simulink subsystem "ButtonStatesRF"  (R2023b)
settings: Solver=VariableStepAuto  StartTime=0.0  StopTime=10.0  RelTol=1e-3
undefined symbols (not defined in the file or in its folder): eStateName
libraries: simulink x5
(part 2 of 2)

== stateflow chart ButtonStatesRF/ButtonStates/buttonFlowChart ==
  state DiagChecker [parallel]
    settings: executionOrder=4
    state CheckHazard [parallel] [commented out]
      state HazardON [commented out]
      state HazardOFF [commented out]
      (default) -> HazardOFF [commented out]
      HazardOFF -> HazardON [commented out] : [hasChangedTo(hazard,true)]
      (junction 4891) -> HazardOFF [commented out]
      (junction 4893) -> (junction 4891) [commented out] : {send(HAZARD, Flow);}
      HazardON -> (junction 4893) [commented out] : [hasChangedTo(ignition,true)]
      (junction 4896) -> (junction 4891) [commented out]
      (junction 4893) -> (junction 4895) [commented out] : [hazard==false]
      (junction 4895) -> (junction 4896) [commented out]
      HazardON -> (junction 4895) [commented out] : [hasChangedTo(hazard,false)]
    state CheckStateReset [parallel]
      settings: executionOrder=1
      state ResetChk
      (default) -> ResetChk
      ResetChk -> (junction 5069) : [hasChangedTo(stateResetFlg,true)]
      (junction 5069) -> (junction 5067)
      (junction 5067) -> ResetChk : {send(RESET, Flow);}
    state CheckVBAT [parallel] [commented out]
      state setHigh [commented out]
        en:
        DASH_RCM_Set_Switch = eButton.pressed;
        en,du:
        setDur = elapsed(sec);
      state setLow [commented out]
        en:
        DASH_RCM_Set_Switch = eButton.released;
      (default) -> DiagChecker.CheckVBAT.setLow [commented out]
      DiagChecker.CheckVBAT.setLow -> DiagChecker.CheckVBAT.setHigh [commented out] : [hasChangedTo(set,true)]
      (junction 4939) -> DiagChecker.CheckVBAT.setLow [commented out]
      (junction 4940) -> (junction 4939) [commented out] : {send(SET,Flow);}
      (junction 4941) -> (junction 4940) [commented out] : [setDur*1000>=BLU_SetDtctMin_Tms && ...
          | setDur*1000 <=BLU_SetDtctMax_Tms ]
      DiagChecker.CheckVBAT.setHigh -> (junction 4941) [commented out] : [hasChangedTo(set,false)]
      (junction 4945) -> (junction 4939) [commented out]
      (junction 4941) -> (junction 4944) [commented out]
      DiagChecker.CheckVBAT.setHigh -> (junction 4942) [commented out] : after(3,sec)
      (junction 4942) -> (junction 4943) [commented out]
      (junction 4944) -> (junction 4943) [commented out] : [setDur*1000>=BLU_LngSetDtctMin_Tms && ...
          | setDur*1000 <=BLU_LngSetDtctMax_Tms ]
      (junction 4943) -> (junction 4945) [commented out] : {send(longSET,Flow);}
      (junction 4944) -> (junction 4947) [commented out]
      (junction 4946) -> (junction 4945) [commented out]
      (junction 4947) -> (junction 4946) [commented out]
    state CheckExit [parallel] [commented out]
      state exitHigh [commented out]
        en:
        DASH_RCM_Exit_Switch = eButton.pressed;
        en,du:
        exitDur = elapsed(sec);
      state exitLow [commented out]
        en:
        DASH_RCM_Exit_Switch = eButton.released;
      (default) -> DiagChecker.CheckExit.exitLow [commented out]
      DiagChecker.CheckExit.exitLow -> DiagChecker.CheckExit.exitHigh [commented out] : [hasChangedTo(exitButton,true)]
      (junction 4966) -> DiagChecker.CheckExit.exitLow [commented out]
      (junction 4967) -> (junction 4966) [commented out] : {send(EXIT,Flow);}
      (junction 4968) -> (junction 4967) [commented out] : [exitDur*1000>=BLU_ExitDtctMin_Tms && ...
          | exitDur*1000 <=BLU_ExitDtctMax_Tms ]
      DiagChecker.CheckExit.exitHigh -> (junction 4968) [commented out] : [hasChangedTo(exitButton,false)]
      (junction 4969) -> (junction 4966) [commented out]
      (junction 4968) -> (junction 4971) [commented out]
      (junction 4970) -> (junction 4969) [commented out] : {send(longEXIT,Flow);}
      (junction 4971) -> (junction 4970) [commented out] : [exitDur*1000>=BLU_LngExitDtctMin_Tms && ...
          | exitDur*1000 <=BLU_LngExitDtctMax_Tms ]
      (junction 4972) -> (junction 4969) [commented out]
      (junction 4971) -> (junction 4973) [commented out]
      (junction 4973) -> (junction 4972) [commented out]
    state CheckCC [parallel] [commented out]
      state setHigh [commented out]
        en:
        DASH_CC_Master_Switch = eButton.pressed;
        en,du:
        ccDur = elapsed(sec);
      state setLow [commented out]
        en:
        DASH_CC_Master_Switch = eButton.released;
      (default) -> DiagChecker.CheckCC.setLow [commented out]
      (junction 4990) -> DiagChecker.CheckCC.setHigh [commented out] : [ECU_CC_Feature==1]
      DiagChecker.CheckCC.setLow -> (junction 4990) [commented out] : [hasChangedTo(cc,true)]
      (junction 4991) -> DiagChecker.CheckCC.setLow [commented out] : {send(CC,Flow);}
      (junction 4992) -> (junction 4991) [commented out] : [ccDur >=BLU_CCDtctMin_Tms && ...
          | ccDur <=BLU_CCDtctMax_Tms ]
      DiagChecker.CheckCC.setHigh -> (junction 4992) [commented out] : [hasChangedTo(cc,false)]
    state CheckResume [parallel] [commented out]
      state setHigh [commented out]
        en:
        DASH_Cc_Resume_Switch = eButton.pressed;
        en,du:
        resumeDur = elapsed(sec)
      state setLow [commented out]
        en:
        DASH_Cc_Resume_Switch = eButton.released;
      (default) -> DiagChecker.CheckResume.setLow [commented out]
      DiagChecker.CheckResume.setLow -> (junction 5002) [commented out] : [hasChangedTo(resume,true)]
      (junction 5002) -> DiagChecker.CheckResume.setHigh [commented out] : [ECU_CC_Feature==1]
      (junction 5003) -> DiagChecker.CheckResume.setLow [commented out] : {send(RESUME,Flow);}
      (junction 5004) -> (junction 5003) [commented out] : [resumeDur >=BLU_ResDtctMin_Tms && ...
          | resumeDur <=BLU_ResDtctMax_Tms ]
      DiagChecker.CheckResume.setHigh -> (junction 5004) [commented out] : [hasChangedTo(resume,false)]
    state CheckCCSet [parallel] [commented out]
      state setHigh [commented out]
        en:
        DASH_Cc_Set_Switch = eButton.pressed;
      state setLow [commented out]
        en:
        DASH_Cc_Set_Switch = eButton.released;
      (default) -> DiagChecker.CheckCCSet.setLow [commented out]
      DiagChecker.CheckCCSet.setLow -> (junction 5014) [commented out] : [hasChangedTo(ccSet,true)]
      (junction 5014) -> DiagChecker.CheckCCSet.setHigh [commented out] : [ECU_CC_Feature==1]
      (junction 5015) -> DiagChecker.CheckCCSet.setLow [commented out] : {send(longCC,Flow);}
      DiagChecker.CheckCCSet.setHigh -> (junction 5015) [commented out] : [duration(set==true,msec) >=BLU_LngCCDtctMin_Tms && ...
          | duration(set==true,msec) <=BLU_LngCCDtctMax_Tms ]
      (junction 5016) -> DiagChecker.CheckCCSet.setLow [commented out] : [hasChangedTo(cc,false)]
          | {send(CCSet,Flow);}
      DiagChecker.CheckCCSet.setHigh -> (junction 5016) [commented out] : [duration(set==true,msec) >=BLU_CCSetDtctMin_Tms && ...
          | duration(set==true,msec) <=BLU_CCSetDtctMax_Tms ]
  state Flow [parallel]
    settings: executionOrder=3
    state ButtonTriggeredMenu
      (history)
      state MainScreen
        state RemoteControMode
          en:
          currentState = eStateName.REMOTE_CONTROL_MODE;
          DASH_RCM_Ready_Switch = eButton.pressed;
        state WaitForButton
          en:
          currentState = eStateName.MAIN_SCREEN;
          QuickSelectorDbg = 0;
        WaitForButton -> RemoteControMode [order 4] : [CCU_RCM_Feature == 1 && ...
            | CCU_RCM_Ready_Status == 1]
        RemoteControMode -> WaitForButton : longEXIT
        (default) -> WaitForButton
        2590 -> WaitForButton
        WaitForButton -> (junction 105) [order 1] : SET
        (junction 105) -> 2593 [order 2]
        (junction 105) -> (junction 107) [order 1] : [ECU_SL_LED == 1 && ...
            | ECU_CC_Feature == 0]
            | {DASH_CC_Master_Switch = eButton.pressed;}
        (junction 107) -> WaitForButton
        WaitForButton -> (junction 60) [order 2] : UP
        (junction 70) -> WaitForButton
        WaitForButton -> (junction 84) [order 3] : DOWN
        (junction 60) -> (junction 70) [order 1] : [ECU_SL_Status == 1  && ...
            | ECU_CC_Feature == 0]
            | {DASH_Cc_Resume_Switch  = eButton.pressed;}
        (junction 84) -> (junction 70) [order 1] : [ECU_SL_Status == 1 && ...
            |  ECU_CC_Feature == 0]
            | {DASH_Cc_Set_Switch  = eButton.pressed;}
        (junction 60) -> 2602 [order 2] : [rideMode != eRideMode.track]
        (junction 90) -> (junction 70)
        (junction 84) -> (junction 88) [order 2]
        (junction 88) -> (junction 90) [order 1] : [rideMode == eRideMode.track]
            | {startLapOrLapTimer();}
        (junction 88) -> 2605 [order 2] : [rideMode != eRideMode.track]
      state Menu
        (history)
        state MainSubMenu
          (history)
          state LapTimer
            en:
            currentState = eStateName.LAP_TIMER;
            state LapTimerEntry
            state GreyedOut
            (default) -> (junction 222)
            (junction 222) -> LapTimerEntry [order 1] : [rideMode == eRideMode.track]
            (junction 222) -> (junction 225) [order 2]
            (junction 225) -> GreyedOut
          state Lap_TimerV2
            (history)
            state LapTimerLoop
              en:
              currentState = eStateName.RESET_LAPS;
            state RoundTimerMenu
              en:
              currentState = eStateName.RESET_LAPS_RND_TMR;
            state ResetLaps
              state ResetLapsMenu
                state Keep
                  en:
                  currentState = eStateName.RESETLAPS_KEEP;
                state Reset
                  en:
                  currentState = eStateName.RESETLAPS_RESET;
                  //updateRoundTimeData();
                (default) -> Flow.ButtonTriggeredMenu.Menu.MainSubMenu.Lap_TimerV2.ResetLaps.ResetLapsMenu.Keep
                Flow.ButtonTriggeredMenu.Menu.MainSubMenu.Lap_TimerV2.ResetLaps.ResetLapsMenu.Keep -> Flow.ButtonTriggeredMenu.Menu.MainSubMenu.Lap_TimerV2.ResetLaps.ResetLapsMenu.Reset [order 1] : DOWN || UP
                Flow.ButtonTriggeredMenu.Menu.MainSubMenu.Lap_TimerV2.ResetLaps.ResetLapsMenu.Reset -> Flow.ButtonTriggeredMenu.Menu.MainSubMenu.Lap_TimerV2.ResetLaps.ResetLapsMenu.Keep [order 1] : DOWN || UP
              Flow.ButtonTriggeredMenu.Menu.MainSubMenu.Lap_TimerV2.ResetLaps.ResetLapsMenu.Keep -> (junction 8239) [order 2] : SET
              (junction 8239) -> 8241
              ResetLapsMenu -> 8241 : EXIT
              (junction 8240) -> 8241
              Flow.ButtonTriggeredMenu.Menu.MainSubMenu.Lap_TimerV2.ResetLaps.ResetLapsMenu.Reset -> (junction 8240) [order 2] : SET
                  | {BLU_FnRLResetRndTmr();}
            (junction 8201) -> 8211
            Flow.ButtonTriggeredMenu.Menu.MainSubMenu.Lap_TimerV2.LapTimerLoop -> 8211 [order 2] : EXIT
            RoundTimerMenu -> (junction 8201) [order 3] : EXIT
            (default) -> (junction 8202)
            (junction 8202) -> Flow.ButtonTriggeredMenu.Menu.MainSubMenu.Lap_TimerV2.LapTimerLoop : {roundTimeCounter = 0;
                | copyRoundTimeData();
                | updateRoundTimeData();}
            8212 -> (junction 8202)
            (junction 8203) -> Flow.ButtonTriggeredMenu.Menu.MainSubMenu.Lap_TimerV2.LapTimerLoop
            (junction 8204) -> (junction 8203) [order 2]
            RoundTimerMenu -> (junction 8204) [order 1] : UP
            (junction 8204) -> (junction 8205) [order 1] : [BLU_RND_TMR_LOOP ==1]
            Flow.ButtonTriggeredMenu.Menu.MainSubMenu.Lap_TimerV2.LapTimerLoop -> (junction 8206) [order 4] : UP
            (junction 8206) -> RoundTimerMenu : [BLU_RND_TMR_LOOP ==1]
                | {BLU_FnLapTmrScrllUP();}
            (junction 8208) -> (junction 8204) [order 2]
            (junction 8205) -> (junction 8207) : {BLU_FnRLRndTmrUp();}
            (junction 8207) -> RoundTimerMenu
            Flow.ButtonTriggeredMenu.Menu.MainSubMenu.Lap_TimerV2.LapTimerLoop -> Flow.ButtonTriggeredMenu.Menu.MainSubMenu.Lap_TimerV2.ResetLaps [order 1] : SET
            8213 -> Flow.ButtonTriggeredMenu.Menu.MainSubMenu.Lap_TimerV2.LapTimerLoop
            Flow.ButtonTriggeredMenu.Menu.MainSubMenu.Lap_TimerV2.LapTimerLoop -> (junction 8210) [order 3] : DOWN
            (junction 8209) -> (junction 8207) : {BLU_FnRLRndTmrDwn();}
            RoundTimerMenu -> (junction 8208) [order 2] : DOWN
            (junction 8208) -> (junction 8209) [order 1] : [BLU_RND_TMR_LOOP ==1]
            (junction 8210) -> RoundTimerMenu : [BLU_RND_TMR_LOOP ==1]
                | {BLU_FnLapTmrScrllDOWN();}
          state MotorCycleSubMenu
            (history)
            state MotorCycleSubMenu1
              (history)
              state RideMode
                en:
                currentState = eStateName.MOTORCYCLE_RIDEMODE;
              state ABS
                en:
                currentState = eStateName.MOTORCYCLE_ABS;
              state MTC
                en:
                currentState = eStateName.MOTORCYCLE_MTC;
              state LaunchControl
                en:
                currentState = eStateName.MOTORCYCLE_LAUNCHCONTROL;
              (default) -> (junction 5718)
              (junction 5718) -> (junction 5719)
              (junction 5719) -> RideMode [order 1] : [variant !=eBikeVariant.duke200]
              RideMode -> 5733 [order 2] : SET
              (junction 5731) -> (junction 5718)
              (junction 5719) -> (junction 5723) [order 2]
              RideMode -> (junction 5720) [order 3] : UP
              (junction 5721) -> RideMode
              5734 -> (junction 5721)
              (junction 5720) -> (junction 5727)
              (junction 5722) -> (junction 5721) : [variant !=eBikeVariant.duke200]
              RideMode -> ABS [order 1] : DOWN
              ABS -> (junction 5722) [order 1] : UP
              (junction 5723) -> ABS
              ABS -> 5735 [order 2] : SET
              (junction 5724) -> ABS
              5736 -> (junction 5724)
              (junction 5728) -> (junction 5724) [order 2]
              MTC -> (junction 5724) [order 2] : UP
              ABS -> (junction 5726) [order 3] : DOWN
              MTC -> (junction 5725) [order 1] : SET
              (junction 5725) -> 5737 : [ABS_TC_MTC_Mode_Feature == eTCMode.TCMode_TC || ...
                  | ABS_TC_MTC_Mode_Feature == eTCMode.TCMode_MTC || ...
                  | ABS_TC_MTC_Mode_Feature == eTCMode.TCMode_TC_Demo || ...
                  | ABS_TC_MTC_Mode_Feature == eTCMode.TCMode_MTC_Demo ]
              5738 -> (junction 5726)
              (junction 5726) -> MTC [order 1] : [ABS_TC_MTC_Mode_Feature !=eTCMode.TCMode_OFF && ...
                  | variant !=eBikeVariant.duke200]
              (junction 5726) -> (junction 5729) [order 2]
              MTC -> (junction 5729) [order 3] : DOWN
              (junction 5728) -> MTC [order 1] : [ABS_TC_MTC_Mode_Feature !=eTCMode.TCMode_OFF]
              (junction 5727) -> (junction 5728) [order 2]
              (junction 5727) -> (junction 5730) [order 1] : [rideMode == eRideMode.rain && ...
                  | ABS_Launch_Control_Feature == 1]
              LaunchControl -> (junction 5728) [order 1] : UP
              (junction 5729) -> LaunchControl [order 1] : [rideMode == eRideMode.rain && ...
                  | ABS_Launch_Control_Feature == 1 && ...
                  | variant !=eBikeVariant.duke200]
              (junction 5729) -> (junction 5732) [order 2]
              LaunchControl -> 5739 [order 2] : SET
              (junction 5730) -> LaunchControl
              (junction 5732) -> (junction 5731)
              LaunchControl -> (junction 5732) [order 3] : DOWN
            state RideModeSubMenu
              (history)
              state RideModeSubMenu1
                (history)
                state Track
                  en:
                  currentState = eStateName.MC_RIDEMODE_TRACK;
                state Street
                  en:
                  currentState = eStateName.MC_RIDEMODE_STREET;
                state Rain
                  en:
                  currentState = eStateName.MC_RIDEMODE_RAIN;
                Track -> (junction 5829) [order 1]
                (junction 5830) -> Track
                (junction 5829) -> (junction 5832) [order 2] : DOWN
                (junction 5833) -> (junction 5830)
                Track -> Street [order 2] : UP
                Street -> Track [order 3] : DOWN
                Street -> Rain [order 2] : UP
                Rain -> Street [order 1] : DOWN
                (junction 5832) -> Rain
                Rain -> (junction 5833) [order 3] : UP
              state TrackCloseThrottle
                en:
                currentState = eStateName.MC_CLOSETHROTTLE;
              state KillSwitch
                en:
                currentState = eStateName.MC_DEACTIVATE_KILLSWITCH;
              state StreetCloseThrottle
                en:
                currentState = eStateName.MC_CLOSETHROTTLE;
              state RainCloseThrottle
                en:
                currentState = eStateName.MC_CLOSETHROTTLE;
              (junction 5829) -> (junction 5781) [order 1] : SET
              (junction 5781) -> (junction 5782) [order 1] : [killSwitch == eSwitch.closed]
              (junction 5782) -> TrackCloseThrottle : {DASH_TC_Mode_Request = 0;
                  | DASH_Drive_Mode_Request = 0;}
              (junction 5781) -> KillSwitch [order 2]
              TrackCloseThrottle -> (junction 5784) [order 2] : after(5,sec)
              TrackCloseThrottle -> (junction 5785) [order 1] : after(500,msec)
              KillSwitch -> (junction 5783) : [after(1,sec)]
              (junction 5784) -> (junction 5783)
              (junction 5785) -> (junction 5784) : [ABS_TC_Mode_Response == 0 && ...
                  | ECU_Drive_Mode_Response == 0]
                  | {rideMode = eRideMode.track;}
              (junction 5783) -> (junction 5790)
              (junction 5787) -> KillSwitch [order 2]
              (junction 5786) -> Street
              5796 -> (junction 5786)
              Street -> (junction 5787) [order 1] : SET
              (junction 5787) -> (junction 5788) [order 1] : [killSwitch == eSwitch.closed]
              (junction 5788) -> StreetCloseThrottle : {DASH_TC_Mode_Request = 0;
                  | DASH_Drive_Mode_Request = 0;}
              StreetCloseThrottle -> (junction 5789) [order 1] : after(500,msec)
              (junction 5794) -> (junction 5787) [order 2]
              (junction 5789) -> (junction 5792) : [ABS_TC_Mode_Response == 0 && ...
                  | ECU_Drive_Mode_Response == 0]
                  | {rideMode = eRideMode.street;}
              StreetCloseThrottle -> (junction 5791) [order 2] : after(5,sec)
              (junction 5791) -> (junction 5790)
              (junction 5792) -> (junction 5791)
              (junction 5790) -> 5797
              RainCloseThrottle -> (junction 5791) [order 2] : after(5,sec)
              (junction 5793) -> (junction 5792) : [ABS_TC_Mode_Response == 2 && ...
                  | ECU_Drive_Mode_Response == 2]
                  | {rideMode = eRideMode.rain;}
              RainCloseThrottle -> (junction 5793) [order 1] : after(500,msec)
              Rain -> (junction 5794) [order 2] : SET
              (junction 5794) -> (junction 5795) [order 1] : [killSwitch == eSwitch.closed]
              (junction 5795) -> RainCloseThrottle : {DASH_TC_Mode_Request = 2;
                  | DASH_Drive_Mode_Request = 2;}
              RideModeSubMenu1 -> 5797 : EXIT
            state ABSSubMenu
              (history)
              state ABSMenu1
                (history)
                state ABSRoad
                  en:
                  currentState = eStateName.ABS_ROAD;
                state ABSSuperMoto
                  en:
                  currentState = eStateName.ABS_SUPERMOTO;
                (default) -> ABSRoad
                ABSRoad -> (junction 5874) [order 1] : DOWN || UP
                ABSSuperMoto -> ABSRoad [order 1] : DOWN || UP
                (junction 5874) -> ABSSuperMoto : [ABS_Mode_Feature == 1]
              state SetABStoRoad
                en:
                DASH_ABS_Mode_Request = eABSMode.road;
              state SetABStoSupermoto
                en:
                DASH_ABS_Mode_Request = eABSMode.supermoto;
              SetABStoRoad -> (junction 5852) [order 1] : after(500,msec)
              ABSRoad -> SetABStoRoad [order 2] : SET
              (junction 5853) -> ABSMenu1
              (junction 5852) -> (junction 5855) : [ABS_ABS_Mode_Response == eABSMode.road]
              5857 -> (junction 5853)
              SetABStoRoad -> (junction 5854) [order 2] : after(5,sec)
              (junction 5855) -> (junction 5854)
              ABSMenu1 -> 5858 : EXIT
              (junction 5854) -> 5858
              SetABStoSupermoto -> (junction 5854) [order 2] : after(5,sec)
              (junction 5856) -> (junction 5855) : [ABS_ABS_Mode_Response == eABSMode.supermoto]
              SetABStoSupermoto -> (junction 5856) [order 1] : after(500,msec)
              ABSSuperMoto -> SetABStoSupermoto [order 2] : SET
            state MTCSubMenu
              (history)
              state MTCMenuOptions
                (history)
                state MTC_Off
                  en:
                  currentState = eStateName.MTCMODE_OFF;
                state MTC_On
                  en:
                  currentState = eStateName.MTCMODE_ON;
                (default) -> MTC_Off
                MTC_Off -> MTC_On [order 1] : DOWN || UP
                MTC_On -> MTC_Off [order 1] : DOWN || UP
              state MTC_Message
                en:
                currentState = eStateName.MTC_KEEP_OFF_PRESS;
              state MTC_Release
                en:
                currentState = eStateName.MTC_OFF_RELEASE;
              state MTC_Message1
                en:
                currentState = eStateName.MTC_KEEP_ON_PRESS;
              state MTC_Release1
                en:
                currentState = eStateName.MTC_ON_RELEASE;
              (junction 5884) -> (junction 5885)
              (junction 5885) -> (junction 5886) [order 1] : [ABS_TC_MTC_Mode_Feature == eTCMode.TCMode_TC || ...
                  | ABS_TC_MTC_Mode_Feature == eTCMode.TCMode_TC_Demo ]
              (junction 5885) -> (junction 5887) [order 2]
              5891 -> (junction 5884)
              (junction 5886) -> (junction 5888) : {currentState = eStateName.MTCMODE_TC;}
              (junction 5887) -> (junction 5888) : {currentState = eStateName.MTCMODE_MTC;}
              (junction 5888) -> MTCMenuOptions
              MTC_Off -> MTC_Message [order 2] : [set]
              MTC_Message -> MTC_Release [order 2] : after(3,sec)
              MTC_Message -> (junction 5889) [order 1] : [!set]
              MTC_Release -> (junction 5890) : [!set]
                  | {DASH_MTC_Off_Switch = eButton.pressed;}
              MTCMenuOptions -> 5892 : EXIT
              (junction 5889) -> 5892
              (junction 5890) -> (junction 5889)
              MTC_Message1 -> (junction 5889) [order 1] : [!set]
              MTC_Release1 -> (junction 5890) : [!set]
                  | {DASH_MTC_Off_Switch = eButton.released;}
              MTC_On -> MTC_Message1 [order 2] : [set]
              MTC_Message1 -> MTC_Release1 [order 2] : after(3,sec)
            state LaunchControlSubMenu
              state LaunchControlSubMenu1
                (history)
                state LaunchControlON
                  en:
                  currentState = eStateName.MC_LAUNCHCONTROL_ON;
                state LaunchControlOFF
                  en:
                  currentState = eStateName.MC_LAUNCHCONTROL_OFF;
                LaunchControlON -> LaunchControlOFF [order 2] : DOWN || UP
                LaunchControlOFF -> LaunchControlON [order 1] : DOWN || UP
              5926 -> LaunchControlON
              LaunchControlON -> (junction 5923) [order 1] : SET
              (junction 5923) -> (junction 5924) : {BLU_FnLaunchControlON();}
              (junction 5925) -> (junction 5924) : {BLU_FnLaunchControlOFF();}
              (junction 5924) -> 5927
              LaunchControlOFF -> (junction 5925) [order 2] : SET
              LaunchControlSubMenu1 -> 5927 : EXIT
            5681 -> 5683
            5680 -> 5684
            5679 -> 5682
            5685 -> MotorCycleSubMenu1
            5686 -> 5687
            5688 -> (junction 5675)
            (junction 5675) -> (junction 5676)
            5689 -> 5690
            5691 -> (junction 5676)
            (junction 5676) -> (junction 5677)
            MotorCycleSubMenu1 -> 5692 : EXIT
            5693 -> 5694
            5695 -> (junction 5677)
            (junction 5677) -> (junction 5678)
            (junction 5678) -> MotorCycleSubMenu1
            5696 -> (junction 5678)
            5697 -> 5698
          state Motorcycle
            en:
            currentState = eStateName.MOTORCYCLE;
          state BikeInfoSubMenu
            (history)
            state BikeInfoSubMenuMain
              (history)
              state WaterTemp
                en:
                currentState = eStateName.WATER_TEMPERATURE;
              state FuelRange
                en:
                currentState = eStateName.FUEL_RANGE;
              state Battery
                en:
                currentState = eStateName.BATTERY;
              state Odometer
                en:
                currentState = eStateName.ODOMETER;
              state Service
                en:
                currentState = eStateName.SERVICE;
              state Warnings
                en:
                currentState = eStateName.WARNINGS;
              (default) -> (junction 5540)
              WaterTemp -> (junction 5541) [order 2] : UP
              (junction 5540) -> WaterTemp
              (junction 5543) -> (junction 5540)
              (junction 5541) -> (junction 5542)
              WaterTemp -> FuelRange [order 1] : DOWN
              FuelRange -> WaterTemp [order 2] : UP
              FuelRange -> Battery [order 1] : DOWN
              Battery -> FuelRange [order 2] : UP
              Battery -> Odometer [order 1] : DOWN
              Odometer -> Battery [order 2] : UP
              Odometer -> Service [order 1] : DOWN
              Service -> Odometer [order 2] : UP
              Service -> Warnings [order 1] : DOWN
              Warnings -> Service [order 1] : UP
              (junction 5542) -> Warnings
              Warnings -> (junction 5543) [order 3] : DOWN
            state WarningsSubMenu
              en:
              currentState = eStateName.WARNINGS_MENU;
            5526 -> (junction 5540)
            5527 -> (junction 5540)
            BikeInfoSubMenuMain -> 5528 : EXIT
            WarningsSubMenu -> (junction 5523) [order 2] : UP
            (junction 5523) -> (junction 5524) : {BLU_FnBIWarningsUp();}
            WarningsSubMenu -> (junction 5542) [order 1] : EXIT
            (junction 5524) -> WarningsSubMenu
            (junction 5525) -> (junction 5524) : {BLU_FnBIWarningsDwn();}
            Warnings -> WarningsSubMenu [order 2] : SET
            WarningsSubMenu -> (junction 5525) [order 3] : DOWN
          state BikeInfo
            en:
            currentState = eStateName.BIKE_INFO;
          state TripInfoSubMenu
            (history)
            state TripInfoSubMenuMain
              (history)
              state TRIP1
                en:
                currentState = eStateName.TRIP_INFO_TRIP_1;
              state TRIP2
                en:
                currentState = eStateName.TRIP_INFO_TRIP_2;
              (default) -> (junction 5602)
              5605 -> (junction 5602)
              (junction 5602) -> TRIP1
              TRIP1 -> 5606 [order 2] : SET
              (junction 5603) -> TRIP1
              TRIP1 -> (junction 5604) [order 1] : DOWN || UP
              5607 -> (junction 5603)
              5608 -> (junction 5604)
              (junction 5604) -> TRIP2
              TRIP2 -> (junction 5603) [order 1] : DOWN || UP
              TRIP2 -> 5609 [order 2] : SET
            state Trip1
              state Trip1SubMenu
                (history)
                state SetAsOverview
                  en:
                  currentState = eStateName.TRIP_1_SET_AS_OVERVIEW;
                state ResetTrip
                  en:
                  currentState = eStateName.TRIP_1_RESET_TRIP;
                Flow.ButtonTriggeredMenu.Menu.MainSubMenu.TripInfoSubMenu.Trip1.Trip1SubMenu.SetAsOverview -> 5635 [order 2] : SET
                    | {BLU_FnTISetAsOvwTrip1();}
                Flow.ButtonTriggeredMenu.Menu.MainSubMenu.TripInfoSubMenu.Trip1.Trip1SubMenu.ResetTrip -> Flow.ButtonTriggeredMenu.Menu.MainSubMenu.TripInfoSubMenu.Trip1.Trip1SubMenu.SetAsOverview [order 1] : DOWN || UP
                Flow.ButtonTriggeredMenu.Menu.MainSubMenu.TripInfoSubMenu.Trip1.Trip1SubMenu.SetAsOverview -> Flow.ButtonTriggeredMenu.Menu.MainSubMenu.TripInfoSubMenu.Trip1.Trip1SubMenu.ResetTrip [order 1] : DOWN || UP
                Flow.ButtonTriggeredMenu.Menu.MainSubMenu.TripInfoSubMenu.Trip1.Trip1SubMenu.ResetTrip -> 5636 [order 2] : SET
                    | {BLU_FnTIResetTrip1();}
              (default) -> (junction 6175)
              (junction 6175) -> Flow.ButtonTriggeredMenu.Menu.MainSubMenu.TripInfoSubMenu.Trip1.Trip1SubMenu.SetAsOverview
              5625 -> 5626
              5627 -> 5628
              Trip1SubMenu -> 5628 : EXIT
            state Trip2
              state Trip2SubMenu
                (history)
                state SetAsOverview
                  en:
                  currentState = eStateName.TRIP_2_SET_AS_OVERVIEW;
                state ResetTrip
                  en:
                  currentState = eStateName.TRIP_2_RESET_TRIP;
                Flow.ButtonTriggeredMenu.Menu.MainSubMenu.TripInfoSubMenu.Trip2.Trip2SubMenu.SetAsOverview -> 8302 [order 1] : SET
                    | {BLU_FnTISetAsOvwTrip2();}
                Flow.ButtonTriggeredMenu.Menu.MainSubMenu.TripInfoSubMenu.Trip2.Trip2SubMenu.SetAsOverview -> Flow.ButtonTriggeredMenu.Menu.MainSubMenu.TripInfoSubMenu.Trip2.Trip2SubMenu.ResetTrip [order 2] : DOWN || UP
                Flow.ButtonTriggeredMenu.Menu.MainSubMenu.TripInfoSubMenu.Trip2.Trip2SubMenu.ResetTrip -> Flow.ButtonTriggeredMenu.Menu.MainSubMenu.TripInfoSubMenu.Trip2.Trip2SubMenu.SetAsOverview [order 1] : DOWN || UP
                Flow.ButtonTriggeredMenu.Menu.MainSubMenu.TripInfoSubMenu.Trip2.Trip2SubMenu.ResetTrip -> 5656 [order 2] : SET
                    | {BLU_FnTIResetTrip2();}
              (default) -> (junction 5644)
              (junction 5644) -> Flow.ButtonTriggeredMenu.Menu.MainSubMenu.TripInfoSubMenu.Trip2.Trip2SubMenu.SetAsOverview
              5645 -> 5646
              5647 -> 5648
              Trip2SubMenu -> 5648 : EXIT
            5576 -> Trip1
            8298 -> 5583
            5579 -> 5580
            5578 -> (junction 5575)
            5581 -> 5582
            TripInfoSubMenuMain -> 5583 : EXIT
            5587 -> 5583
            (junction 5575) -> TripInfoSubMenuMain
            5586 -> (junction 5575)
            5584 -> 5585
            5588 -> Trip2
          state TripInfo
            en:
            currentState = eStateName.TRIP_INFO;
          state CruiseCtrlSubMenu
            (history)
            note: Invisible when ECU_CC_Feature == 0
            note: Invisible when ECU_CC_Feature == 1
            state Mode
              en:
              currentState = eStateName.MODE_CC;
            state SpeedLimiter
              en:
              currentState = eStateName.SPEED_LIMITER;
            state SpeedLimiterSubMenu
              (history)
              state SL_ON
                en:
                currentState = eStateName.SPEED_LIMITER_ON;
              state SL_OFF
                en:
                currentState = eStateName.SPEED_LIMITER_OFF;
              (default) -> SL_ON
              SL_ON -> SL_OFF [order 1] : DOWN || UP
              SL_OFF -> SL_ON [order 1] : DOWN || UP
            state SpeedLimiterSelChk
              state DashSLONCheck
                en:
                currentState = eStateName.SPEED_LIMITER_ONCHECK;
              state DashSLOFFCheck
                en:
                currentState = eStateName.SPEED_LIMITER_OFFCHECK;
            state ModeSubMenu
              (history)
              state ModeCC_CruiseControlSel
                en:
                currentState = eStateName.CRUISE_CONTROL_MODE;
              state SpeedLimiterMode
                en:
                currentState = eStateName.SPEED_LIMITER_MODE;
              (default) -> ModeCC_CruiseControlSel
              ModeCC_CruiseControlSel -> SpeedLimiterMode [order 1] : DOWN || UP
              SpeedLimiterMode -> ModeCC_CruiseControlSel [order 1] : DOWN || UP
            state ModeCCSelChk
              state DashCruiseCtrlChck
                en:
                currentState = eStateName.CRUISE_CONTROL_CHECK;
              state DashSpeedLmtrChck
                en:
                currentState = eStateName.SPEED_LIMITER_MODE_CHECK;
            5967 -> (junction 5954)
            (default) -> (junction 5954)
            (junction 5954) -> SpeedLimiter [order 2]
            (junction 5954) -> Mode [order 1] : [ECU_CC_Feature == 1]
            5968 -> (junction 5954)
            SpeedLimiter -> SpeedLimiterSubMenu [order 1] : SET
            (junction 5960) -> SpeedLimiter
            SpeedLimiter -> 5969 [order 2] : EXIT
            Mode -> (junction 5959) [order 1] : EXIT
            (junction 5957) -> Mode
            Mode -> ModeSubMenu [order 2] : SET
            (junction 5956) -> (junction 5955)
            (junction 5955) -> (junction 5958)
            (junction 5958) -> (junction 5957)
            (junction 5959) -> 5969
            ModeCCSelChk -> (junction 5955) [order 2] : [after(5,sec)]
            ModeCCSelChk -> (junction 5956) [order 1] : EXIT
            ModeSubMenu -> (junction 5957) : EXIT
            (junction 5963) -> (junction 5958)
            SpeedLimiterSubMenu -> (junction 5960) : EXIT
            (junction 5961) -> (junction 5960)
            (junction 5962) -> (junction 5961)
            (junction 5964) -> (junction 5960)
            SpeedLimiterSelChk -> (junction 5961) [order 2] : [after(5,sec)]
            SpeedLimiterSelChk -> (junction 5962) [order 1] : EXIT
            ModeCC_CruiseControlSel -> DashCruiseCtrlChck [order 2] : SET
                | {DASH_SL_Switch =1;}
            SL_ON -> DashSLONCheck [order 2] : SET
                | {DASH_SL_Switch =1;}
            DashCruiseCtrlChck -> (junction 5963) : [ ECU_SL_Status == 0]
                | {BLU_FnCCSwtONCrsCtrl();}
            DashSLONCheck -> (junction 5964) : [ ECU_SL_Status == 1]
                | {BLU_FnCCSwtONSpdLmtr();}
            (junction 5966) -> (junction 5963)
            (junction 5965) -> (junction 5964)
            SL_OFF -> DashSLOFFCheck [order 2] : SET
                | {DASH_SL_Switch =1;}
            SpeedLimiterMode -> DashSpeedLmtrChck [order 2] : SET
                | {DASH_SL_Switch =1;}
            DashSLOFFCheck -> (junction 5965) : [ ECU_SL_Status == 0]
                | {BLU_FnCCSwtOFFSpdLmtr();}
            DashSpeedLmtrChck -> (junction 5966) : [ ECU_SL_Status == 1]
                | {BLU_FnCCSwtONSpdLmtr();}
          state CruiseControlMain
            en:
            currentState = eStateName.CRUISE_CONTROL;
          state NavigationVolSubMenu
            (history)
            state NavGroup
              (history)
              state Volume
                en:
                currentState = eStateName.NAVIGATION_VOLUME;
              state Navigation
                en:
                currentState = eStateName.NAVIGATION;
              (default) -> Volume
              Volume -> Navigation [order 1] : DOWN || UP
              Navigation -> Volume [order 1] : DOWN || UP
            state VolumeSubMenu
              en:
              currentState = eStateName.NAVIGATION_VOL_SUB_MN;
            state NavigationSubMenu
              (history)
              state Nav_ON
                en:
                currentState = eStateName.NAVIGATION_ON;
              state Nav_OFF
                en:
                currentState = eStateName.NAVIGATION_OFF;
              (default) -> Nav_ON
              Nav_ON -> Nav_OFF [order 1] : DOWN || UP
              Nav_OFF -> Nav_ON [order 1] : DOWN || UP
            6046 -> (junction 6039)
            VolumeSubMenu -> (junction 6037) [order 2] : UP
            (junction 6037) -> (junction 6038) : {BLU_FnNavVolUp();}
            (junction 6038) -> VolumeSubMenu
            (junction 6039) -> NavGroup
            (junction 6040) -> (junction 6038) : {BLU_FnNavVolDwn();}
            Volume -> VolumeSubMenu [order 2] : SET
            6047 -> (junction 6039)
            VolumeSubMenu -> (junction 6040) [order 3] : DOWN
            VolumeSubMenu -> (junction 6041) [order 1] : EXIT
            (junction 6041) -> NavGroup
            (junction 6042) -> (junction 6041)
            NavigationSubMenu -> (junction 6041) : EXIT
            (junction 6044) -> (junction 6042)
            NavGroup -> 6048 : EXIT
            Navigation -> NavigationSubMenu [order 2] : SET
            Nav_ON -> (junction 6043) [order 2] : SET
            (junction 6043) -> (junction 6044) : {navigationStatus = eFeatureStatus.enabled;
                | BLU_FnNavigationStatus(navigationStatus);}
            (junction 6045) -> (junction 6044) : {navigationStatus = eFeatureStatus.disabled;
                | BLU_FnNavigationStatus(navigationStatus);}
            Nav_OFF -> (junction 6045) [order 2] : SET
          state NavigationMain
            en:
            currentState = eStateName.MAIN_NAVIGATION;
            state NavigationGreyedOut
            state NavigationVisible
            (default) -> (junction 1075)
            (junction 1075) -> NavigationGreyedOut [order 1] : [CCU_BT_Status == 0]
                | % || rideMode == eRideMode.track]
            (junction 1075) -> (junction 1080) [order 2]
            (junction 1080) -> NavigationVisible
          state HeatedGripsMain
            en:
            currentState = eStateName.HEATED_GRIPS;
          state HeatedGripsSubMenu
            (history)
            state HeatedGripsMenuGrp
              (history)
              state HeatedGripsOFF
                en:
                currentState = eStateName.HEATED_GRIPS_OFF;
                HeatedGripsMode = eHeatedGripsMode.OFF;
              state HeatedGripsMAXIMUM
                en:
                currentState = eStateName.HEATED_GRIPS_MAXIMUM;
                HeatedGripsMode = eHeatedGripsMode.MAXIMUM;
              state HeatedGripsMEDIUM
                en:
                currentState = eStateName.HEATED_GRIPS_MEDIUM;
                HeatedGripsMode = eHeatedGripsMode.MEDIUM;
              state HeatedGripsMINIMUM
                en:
                currentState = eStateName.HEATED_GRIPS_MINIMUM;
                HeatedGripsMode = eHeatedGripsMode.MINIMUM;
              HeatedGripsOFF -> (junction 6103) [order 2] : UP
              (junction 6103) -> (junction 6105)
              (junction 6104) -> HeatedGripsOFF
              (junction 6106) -> (junction 6104)
              HeatedGripsOFF -> HeatedGripsMAXIMUM [order 1] : DOWN
              HeatedGripsMAXIMUM -> HeatedGripsOFF [order 1] : UP
              HeatedGripsMAXIMUM -> HeatedGripsMEDIUM [order 2] : DOWN
              HeatedGripsMEDIUM -> HeatedGripsMAXIMUM [order 1] : UP
              HeatedGripsMEDIUM -> HeatedGripsMINIMUM [order 2] : DOWN
              HeatedGripsMINIMUM -> HeatedGripsMEDIUM [order 1] : UP
              (junction 6105) -> HeatedGripsMINIMUM
              HeatedGripsMINIMUM -> (junction 6106) [order 2] : DOWN
            6093 -> (junction 6104)
            6094 -> (junction 6104)
            HeatedGripsMenuGrp -> 6095 [order 1] : EXIT
            (junction 6092) -> 6095 : {BLU_FnSetHeatedGripsMode(HeatedGripsMode);}
            HeatedGripsMenuGrp -> (junction 6092) [order 2] : SET
          state AudioMain
            en:
            currentState = eStateName.AUDIO;
            state AudioVisible
            state AudioGreyedOut
            (default) -> (junction 1063)
            (junction 1063) -> AudioVisible [order 1] : [CCU_BT_Status == 1]
            (junction 1063) -> (junction 1069) [order 2]
            (junction 1069) -> AudioGreyedOut
          state AudioSubMenu
            (history)
            state AudioMain
              en:
              currentState = eStateName.AUDIO_SUB_MENU;
            Flow.ButtonTriggeredMenu.Menu.MainSubMenu.AudioSubMenu.AudioMain -> (junction 6130) [order 1] : SET
            (junction 6130) -> (junction 6131) : {BLU_FnAudioTglPlayPause();}
            (junction 6131) -> (junction 6133)
            6142 -> (junction 6136)
            Flow.ButtonTriggeredMenu.Menu.MainSubMenu.AudioSubMenu.AudioMain -> (junction 6132) [order 2] : longUP
            (junction 6132) -> (junction 6133) : {BLU_FnAudioVolUp();}
            (junction 6133) -> (junction 6135)
            Flow.ButtonTriggeredMenu.Menu.MainSubMenu.AudioSubMenu.AudioMain -> (junction 6134) [order 3] : longDOWN
            (junction 6134) -> (junction 6135) : {BLU_FnAudioVolDwn();}
            6143 -> (junction 6136)
            (junction 6136) -> Flow.ButtonTriggeredMenu.Menu.MainSubMenu.AudioSubMenu.AudioMain
            (junction 6135) -> (junction 6138)
            Flow.ButtonTriggeredMenu.Menu.MainSubMenu.AudioSubMenu.AudioMain -> (junction 6137) [order 4] : UP
            (junction 6137) -> (junction 6138) : {BLU_FnAudioNextTrack();}
            (junction 6138) -> (junction 6140)
            Flow.ButtonTriggeredMenu.Menu.MainSubMenu.AudioSubMenu.AudioMain -> 6144 [order 6] : EXIT
            Flow.ButtonTriggeredMenu.Menu.MainSubMenu.AudioSubMenu.AudioMain -> (junction 6139) [order 5] : DOWN
            (junction 6139) -> (junction 6140) : {BLU_FnAudioPrevTrack();}
            (junction 6140) -> (junction 6141)
            (junction 6141) -> Flow.ButtonTriggeredMenu.Menu.MainSubMenu.AudioSubMenu.AudioMain
          state SettingsMain
            en:
            currentState = eStateName.SETTINGS;
            state SettingsGreyedOut
            state SettingsMenu
            (default) -> (junction 1245)
            (junction 1245) -> SettingsGreyedOut [order 1] : [vehSpeed>2.5]
            (junction 1245) -> (junction 1249) [order 2]
            (junction 1249) -> SettingsMenu
            SettingsMenu -> 3011 [order 1] : [duration(up && down,msec)>=3000]
          state SettingsSubMenu
            (history)
            state SettingsMenuScreens
              (history)
              state Favourites
                en:
                currentState = eStateName.FAVOURITES;
              state QuickSelector
                en:
                currentState = eStateName.QUICK_SELECTOR;
              state Connectivity
                en:
                currentState = eStateName.CONNECTIVITY;
              state QuickShifter
                en:
                currentState = eStateName.QUICK_SHIFTER;
              state ShiftLight
                en:
                currentState = eStateName.SHIFT_LIGHT;
              state ClockDate
                en:
                currentState = eStateName.CLOCK_DATE_MN;
              state Units
                en:
                currentState = eStateName.UNITS;
              state Language
                en:
                currentState = eStateName.LANGUAGE;
              state HeatedGripsSettingsMenu
                en:
                currentState = eStateName.HEATED_GRIPS_SETTINGS_MN;
              state ExtraFunctions
                en:
                currentState = eStateName.EXTRA_FUNCTIONS;
              (default) -> (junction 6255)
              Favourites -> 6277 [order 1] : SET
              (junction 6255) -> Favourites
              (junction 6275) -> (junction 6255)
              6278 -> Favourites
              Favourites -> (junction 6256) [order 3] : UP
              (junction 6256) -> (junction 6274)
              Favourites -> QuickSelector [order 2] : DOWN
              QuickSelector -> Favourites [order 2] : UP
              6279 -> QuickSelector
              QuickSelector -> 6280 [order 1] : SET
              (junction 6257) -> QuickSelector
              (junction 6258) -> (junction 6257) [order 2] : UP
              (junction 6260) -> (junction 6257) [order 2]
              QuickSelector -> (junction 6259) [order 3] : DOWN
              6281 -> Connectivity
              Connectivity -> (junction 6258) [order 1]
              (junction 6258) -> 6282 [order 1] : SET
              (junction 6259) -> Connectivity [order 1] : [CCU_Status == eCCUStatus.installed]
              (junction 6259) -> (junction 6263) [order 2]
              Connectivity -> (junction 6263) [order 2] : DOWN
              (junction 6260) -> Connectivity [order 1] : [CCU_Status == eCCUStatus.installed]
              (junction 6261) -> (junction 6260) [order 1] : UP
              (junction 6262) -> (junction 6260) [order 2]
              6283 -> QuickShifter
              QuickShifter -> (junction 6261) [order 1]
              (junction 6261) -> 6284 [order 2] : SET
              (junction 6262) -> QuickShifter [order 1] : [ECU_Quickshifter_Feature != 0 && ...
                  | variant !=eBikeVariant.duke200]
              (junction 6263) -> QuickShifter [order 1] : [ECU_Quickshifter_Feature != 0 && ...
                  | variant !=eBikeVariant.duke200]
              (junction 6264) -> (junction 6262)
              (junction 6263) -> (junction 6265) [order 2]
              QuickShifter -> (junction 6265) [order 2] : DOWN
              Flow.ButtonTriggeredMenu.Menu.MainSubMenu.SettingsSubMenu.SettingsMenuScreens.ShiftLight -> (junction 6264) [order 2] : UP
              Flow.ButtonTriggeredMenu.Menu.MainSubMenu.SettingsSubMenu.SettingsMenuScreens.ShiftLight -> 6285 [order 3] : SET
              (junction 6265) -> Flow.ButtonTriggeredMenu.Menu.MainSubMenu.SettingsSubMenu.SettingsMenuScreens.ShiftLight
              Flow.ButtonTriggeredMenu.Menu.MainSubMenu.SettingsSubMenu.SettingsMenuScreens.ShiftLight -> (junction 6266) [order 1] : DOWN
              6286 -> Flow.ButtonTriggeredMenu.Menu.MainSubMenu.SettingsSubMenu.SettingsMenuScreens.ShiftLight
              (junction 6266) -> ClockDate
              (junction 6267) -> Flow.ButtonTriggeredMenu.Menu.MainSubMenu.SettingsSubMenu.SettingsMenuScreens.ShiftLight [order 1] : UP
              ClockDate -> (junction 6267) [order 1]
              (junction 6267) -> 6287 [order 2] : SET
              6288 -> ClockDate
              ClockDate -> Units [order 2] : DOWN
              Units -> ClockDate [order 2] : UP
              6289 -> (junction 6268)
              Units -> 6290 [order 3] : SET
              (junction 6268) -> Units
              (junction 6271) -> (junction 6268) [order 2]
              Units -> (junction 6269) [order 1] : DOWN
              Language -> Units [order 1] : UP
              Language -> 6291 [order 3] : SET
              (junction 6269) -> Language [order 1] : [variant !=eBikeVariant.duke200]
              (junction 6270) -> Language
              (junction 6271) -> (junction 6270) [order 1] : [variant !=eBikeVariant.duke200]
              (junction 6269) -> (junction 6274) [order 2]
              HeatedGripsSettingsMenu -> (junction 6271) [order 1] : UP
              6292 -> (junction 6270)
              (junction 6273) -> (junction 6271) [order 2]
              Language -> (junction 6272) [order 2] : DOWN
              HeatedGripsSettingsMenu -> 6293 [order 3] : SET
              (junction 6272) -> HeatedGripsSettingsMenu [order 1] : [Heated_Grips]
              (junction 6272) -> ExtraFunctions [order 2]
              (junction 6273) -> HeatedGripsSettingsMenu [order 1] : [Heated_Grips && ...
                  | variant !=eBikeVariant.duke200]
              (junction 6276) -> (junction 6273)
              HeatedGripsSettingsMenu -> ExtraFunctions [order 2] : DOWN
              6294 -> HeatedGripsSettingsMenu
              (junction 6274) -> ExtraFunctions
              ExtraFunctions -> (junction 8379) [order 2] : [CCU_Connect==1]
              (junction 8379) -> 6295 : SET
              ExtraFunctions -> (junction 6275) [order 3] : DOWN
              ExtraFunctions -> (junction 6276) [order 1] : UP
              6296 -> ExtraFunctions
            state FavoritesSubMenu
              (history)
              state FavoritesSlotsMenu
                (history)
                state FavoritesSlot1
                  en:
                  currentState = eStateName.FAVORITES_SLOT_1;
                state FavoritesSlot2
                  en:
                  currentState = eStateName.FAVORITES_SLOT_2;
                state FavoritesSlot3
                  en:
                  currentState = eStateName.FAVORITES_SLOT_3;
                state FavoritesSlot4
                  en:
                  currentState = eStateName.FAVORITES_SLOT_4;
                (default) -> (junction 6401)
                (junction 6401) -> FavoritesSlot1
                (junction 6404) -> (junction 6401)
                FavoritesSlot1 -> (junction 6402) [order 3] : UP
                (junction 6402) -> (junction 6403)
                FavoritesSlot1 -> FavoritesSlot2 [order 1] : DOWN
                FavoritesSlot2 -> FavoritesSlot1 [order 2] : UP
                FavoritesSlot2 -> FavoritesSlot3 [order 3] : DOWN
                FavoritesSlot3 -> FavoritesSlot2 [order 1] : UP
                FavoritesSlot3 -> FavoritesSlot4 [order 2] : DOWN
                FavoritesSlot4 -> FavoritesSlot3 [order 1] : UP
                (junction 6403) -> FavoritesSlot4
                FavoritesSlot4 -> (junction 6404) [order 3] : DOWN
              state Slot1Menu
                (history)
                state Slot1FavoritesMenu
                  (history)
                  state Slot1TripDistance1
                    en:
                    currentState = eStateName.FAVORITES_TRIP_DISTANCE_1;
                  state Slot1AverageSpeed1
                    en:
                    currentState = eStateName.FAVORITES_AVERAGE_SPEED_1;
                  state Slot1AverageFuelCons1
                    en:
                    currentState = eStateName.FAVORITES_AVERAGE_FUEL_CONS_1;
                  state Slot1TripTime1
                    en:
                    currentState = eStateName.FAVORITES_TRIP_TIME_1;
                  state Slot1AverageTripDistance2
                    en:
                    currentState = eStateName.FAVORITES_TRIP_DISTANCE_2;
                  state Slot1AverageSpeed2
                    en:
                    currentState = eStateName.FAVORITES_AVERAGE_SPEED_2;
                  state Slot1AverageFuelCons2
                    en:
                    currentState = eStateName.FAVORITES_AVERAGE_FUEL_CONS_2;
                  state Slot1TripTime2
                    en:
                    currentState = eStateName.FAVORITES_TRIP_TIME_2;
                  state Slot1FuelRange
                    en:
                    currentState = eStateName.FAVORITES_FUEL_RANGE;
                  state Slot1Date
                    en:
                    currentState = eStateName.FAVORITES_DATE;
                  state Slot1Battery
                    en:
                    currentState = eStateName.FAVORITES_BATTERY;
                  state Slot1WaterTemperature
                    en:
                    currentState = eStateName.FAVORITES_WATER_TEMPERATURE;
                  state Slot1Odometer
                    en:
                    currentState = eStateName.FAVORITES_ODOMETER;
                  state Slot1Connectivity
                    en:
                    currentState = eStateName.FAVORITES_CONNECTIVITY;
                  (default) -> (junction 6432)
                  (junction 6432) -> Flow.ButtonTriggeredMenu.Menu.MainSubMenu.SettingsSubMenu.FavoritesSubMenu.Slot1Menu.Slot1FavoritesMenu.Slot1TripDistance1
                  Flow.ButtonTriggeredMenu.Menu.MainSubMenu.SettingsSubMenu.FavoritesSubMenu.Slot1Menu.Slot1FavoritesMenu.Slot1TripDistance1 -> (junction 6433) [order 2] : UP
                  (junction 6435) -> (junction 6432)
                  (junction 6433) -> (junction 6434)
                  Flow.ButtonTriggeredMenu.Menu.MainSubMenu.SettingsSubMenu.FavoritesSubMenu.Slot1Menu.Slot1FavoritesMenu.Slot1TripDistance1 -> Flow.ButtonTriggeredMenu.Menu.MainSubMenu.SettingsSubMenu.FavoritesSubMenu.Slot1Menu.Slot1FavoritesMenu.Slot1AverageSpeed1 [order 1] : DOWN
                  Flow.ButtonTriggeredMenu.Menu.MainSubMenu.SettingsSubMenu.FavoritesSubMenu.Slot1Menu.Slot1FavoritesMenu.Slot1AverageSpeed1 -> Flow.ButtonTriggeredMenu.Menu.MainSubMenu.SettingsSubMenu.FavoritesSubMenu.Slot1Menu.Slot1FavoritesMenu.Slot1TripDistance1 [order 1] : UP
                  Flow.ButtonTriggeredMenu.Menu.MainSubMenu.SettingsSubMenu.FavoritesSubMenu.Slot1Menu.Slot1FavoritesMenu.Slot1AverageSpeed1 -> Flow.ButtonTriggeredMenu.Menu.MainSubMenu.SettingsSubMenu.FavoritesSubMenu.Slot1Menu.Slot1FavoritesMenu.Slot1AverageFuelCons1 [order 2] : DOWN
                  Flow.ButtonTriggeredMenu.Menu.MainSubMenu.SettingsSubMenu.FavoritesSubMenu.Slot1Menu.Slot1FavoritesMenu.Slot1AverageFuelCons1 -> Flow.ButtonTriggeredMenu.Menu.MainSubMenu.SettingsSubMenu.FavoritesSubMenu.Slot1Menu.Slot1FavoritesMenu.Slot1AverageSpeed1 [order 1] : UP
                  Flow.ButtonTriggeredMenu.Menu.MainSubMenu.SettingsSubMenu.FavoritesSubMenu.Slot1Menu.Slot1FavoritesMenu.Slot1AverageFuelCons1 -> Flow.ButtonTriggeredMenu.Menu.MainSubMenu.SettingsSubMenu.FavoritesSubMenu.Slot1Menu.Slot1FavoritesMenu.Slot1TripTime1 [order 2] : DOWN
                  Flow.ButtonTriggeredMenu.Menu.MainSubMenu.SettingsSubMenu.FavoritesSubMenu.Slot1Menu.Slot1FavoritesMenu.Slot1TripTime1 -> Flow.ButtonTriggeredMenu.Menu.MainSubMenu.SettingsSubMenu.FavoritesSubMenu.Slot1Menu.Slot1FavoritesMenu.Slot1AverageFuelCons1 [order 1] : UP
                  Flow.ButtonTriggeredMenu.Menu.MainSubMenu.SettingsSubMenu.FavoritesSubMenu.Slot1Menu.Slot1FavoritesMenu.Slot1TripTime1 -> Flow.ButtonTriggeredMenu.Menu.MainSubMenu.SettingsSubMenu.FavoritesSubMenu.Slot1Menu.Slot1FavoritesMenu.Slot1AverageTripDistance2 [order 2] : DOWN
                  Flow.ButtonTriggeredMenu.Menu.MainSubMenu.SettingsSubMenu.FavoritesSubMenu.Slot1Menu.Slot1FavoritesMenu.Slot1AverageTripDistance2 -> Flow.ButtonTriggeredMenu.Menu.MainSubMenu.SettingsSubMenu.FavoritesSubMenu.Slot1Menu.Slot1FavoritesMenu.Slot1TripTime1 [order 1] : UP
                  Flow.ButtonTriggeredMenu.Menu.MainSubMenu.SettingsSubMenu.FavoritesSubMenu.Slot1Menu.Slot1FavoritesMenu.Slot1AverageTripDistance2 -> Flow.ButtonTriggeredMenu.Menu.MainSubMenu.SettingsSubMenu.FavoritesSubMenu.Slot1Menu.Slot1FavoritesMenu.Slot1AverageSpeed2 [order 2] : DOWN
                  Flow.ButtonTriggeredMenu.Menu.MainSubMenu.SettingsSubMenu.FavoritesSubMenu.Slot1Menu.Slot1FavoritesMenu.Slot1AverageSpeed2 -> Flow.ButtonTriggeredMenu.Menu.MainSubMenu.SettingsSubMenu.FavoritesSubMenu.Slot1Menu.Slot1FavoritesMenu.Slot1AverageTripDistance2 [order 1] : UP
                  Flow.ButtonTriggeredMenu.Menu.MainSubMenu.SettingsSubMenu.FavoritesSubMenu.Slot1Menu.Slot1FavoritesMenu.Slot1AverageSpeed2 -> Flow.ButtonTriggeredMenu.Menu.MainSubMenu.SettingsSubMenu.FavoritesSubMenu.Slot1Menu.Slot1FavoritesMenu.Slot1AverageFuelCons2 [order 2] : DOWN
                  Flow.ButtonTriggeredMenu.Menu.MainSubMenu.SettingsSubMenu.FavoritesSubMenu.Slot1Menu.Slot1FavoritesMenu.Slot1AverageFuelCons2 -> Flow.ButtonTriggeredMenu.Menu.MainSubMenu.SettingsSubMenu.FavoritesSubMenu.Slot1Menu.Slot1FavoritesMenu.Slot1AverageSpeed2 [order 1] : UP
                  Flow.ButtonTriggeredMenu.Menu.MainSubMenu.SettingsSubMenu.FavoritesSubMenu.Slot1Menu.Slot1FavoritesMenu.Slot1AverageFuelCons2 -> Flow.ButtonTriggeredMenu.Menu.MainSubMenu.SettingsSubMenu.FavoritesSubMenu.Slot1Menu.Slot1FavoritesMenu.Slot1TripTime2 [order 2] : DOWN
                  Flow.ButtonTriggeredMenu.Menu.MainSubMenu.SettingsSubMenu.FavoritesSubMenu.Slot1Menu.Slot1FavoritesMenu.Slot1TripTime2 -> Flow.ButtonTriggeredMenu.Menu.MainSubMenu.SettingsSubMenu.FavoritesSubMenu.Slot1Menu.Slot1FavoritesMenu.Slot1AverageFuelCons2 [order 1] : UP
                  Flow.ButtonTriggeredMenu.Menu.MainSubMenu.SettingsSubMenu.FavoritesSubMenu.Slot1Menu.Slot1FavoritesMenu.Slot1TripTime2 -> Flow.ButtonTriggeredMenu.Menu.MainSubMenu.SettingsSubMenu.FavoritesSubMenu.Slot1Menu.Slot1FavoritesMenu.Slot1FuelRange [order 2] : DOWN
                  Flow.ButtonTriggeredMenu.Menu.MainSubMenu.SettingsSubMenu.FavoritesSubMenu.Slot1Menu.Slot1FavoritesMenu.Slot1FuelRange -> Flow.ButtonTriggeredMenu.Menu.MainSubMenu.SettingsSubMenu.FavoritesSubMenu.Slot1Menu.Slot1FavoritesMenu.Slot1TripTime2 [order 1] : UP
                  Flow.ButtonTriggeredMenu.Menu.MainSubMenu.SettingsSubMenu.FavoritesSubMenu.Slot1Menu.Slot1FavoritesMenu.Slot1FuelRange -> Flow.ButtonTriggeredMenu.Menu.MainSubMenu.SettingsSubMenu.FavoritesSubMenu.Slot1Menu.Slot1FavoritesMenu.Slot1Date [order 2] : DOWN
                  Flow.ButtonTriggeredMenu.Menu.MainSubMenu.SettingsSubMenu.FavoritesSubMenu.Slot1Menu.Slot1FavoritesMenu.Slot1Date -> Flow.ButtonTriggeredMenu.Menu.MainSubMenu.SettingsSubMenu.FavoritesSubMenu.Slot1Menu.Slot1FavoritesMenu.Slot1FuelRange [order 1] : UP